simulink library "inp"  (Simulink 8.2)
settings: Solver=ode45  SolverMode=SingleTasking  StartTime=0.0  StopTime=10.0  RelTol=1e-3
undefined symbols (not defined in the file or in its folder): C_TICK_TIME_S, ENUM_FAULTSTATE_FAIL, ENUM_FAULTSTATE_INDETERMINATE, ENUM_FAULTSTATE_PASS, ENUM_KEYSWITCH_ON, FALSE, KINP_ABS1CANCommuOk_flg_ovrdflg, KINP_ABS1CANCommuOk_flg_ovrdval, KINP_ABS1CANPeriodTime_s, KINP_ABS1CANRolCntOk_flg_ovrdflg, KINP_ABS1CANRolCntOk_flg_ovrdval, KINP_ABS2CANChkSumOk_flg_ovrdflg, KINP_ABS2CANChkSumOk_flg_ovrdval, KINP_ABS2CANCommuOk_flg_ovrdflg, KINP_ABS2CANCommuOk_flg_ovrdval, KINP_ABS2CANPeriodTime_s, KINP_ABS2CANRolCntOk_flg_ovrdflg, KINP_ABS2CANRolCntOk_flg_ovrdval, KINP_ABS3CANCommuOk_flg_ovrdflg, KINP_ABS3CANCommuOk_flg_ovrdval, KINP_ABS4CANCommuOk_flg_ovrdflg, KINP_ABS4CANCommuOk_flg_ovrdval, KINP_ABSActive_flg_defval, KINP_ABSActive_flg_invalid, KINP_ABSActive_flg_ovrdflg, KINP_ABSActive_flg_ovrdval, KINP_ABSActive_flg_setvalid, KINP_ABSActive_flg_srvcflg, KINP_ABSActive_flg_srvcval, KINP_ABSCANAlive_flg_ovrdflg, KINP_ABSCANAlive_flg_ovrdval, KINP_ABSCANCommuOk_flg_ovrdflg, KINP_ABSCANCommuOk_flg_ovrdval, KINP_ABSCANValidOk_flg_ovrdflg, KINP_ABSCANValidOk_flg_ovrdval, KINP_ABSFault_flg_defval, KINP_ABSFault_flg_invalid, KINP_ABSFault_flg_ovrdflg, KINP_ABSFault_flg_ovrdval, KINP_ABSFault_flg_setvalid, KINP_ABSFault_flg_srvcflg, KINP_ABSFault_flg_srvcval, KINP_AC1CANCommuOk_flg_ovrdflg, KINP_AC1CANCommuOk_flg_ovrdval, KINP_ACCANAlive_flg_ovrdflg, KINP_ACCANAlive_flg_ovrdval, KINP_ACCANCommuOk_flg_ovrdflg, KINP_ACCANCommuOk_flg_ovrdval, KINP_ACCANValidOk_flg_ovrdflg, KINP_ACCANValidOk_flg_ovrdval, KINP_ACCCancelH_flg_defval, KINP_ACCCancelH_flg_invalid, KINP_ACCCancelH_flg_ovrdflg, KINP_ACCCancelH_flg_ovrdval, KINP_ACCCancelH_flg_setvalid, KINP_ACCCancelH_flg_srvcflg, KINP_ACCCancelH_flg_srvcval, KINP_AccelPedalPosn_pct_defval, KINP_AccelPedalPosn_pct_gain, KINP_AccelPedalPosn_pct_invalid ... (+1866)
libraries: inp x192, hcu_lib x34, simulink x33
(part 2 of 21)

== system inp/INP/BCM_signals ==
  BHAL_outputs : Inport  OutDataTypeStr=Bus: BHAL_outputs
  CANvalid_bus : Inport  Port=2
  AnyDoorOpen : Subsystem
      settings: RTWSystemCode=Inline
  Bus Selector1 : BusSelector  OutputSignals=VHAL_KeySwitchStatus_enum
  Bus Selector{2,5,7..8,10..11,13,15,17,19} (10 alike) : BusSelector  OutputSignals=VINP_CANMsgValidBCM1_flg
  Bus Selector12 : BusSelector  OutputSignals=VHAL_LRDoorOpen_flg
  Bus Selector14 : BusSelector  OutputSignals=VHAL_RRDoorOpen_flg
  Bus Selector16 : BusSelector  OutputSignals=VHAL_TailGateOpen_flg
  Bus Selector18 : BusSelector  OutputSignals=VHAL_AnyDoorOpen_flg
  Bus Selector20 : BusSelector  OutputSignals=VHAL_HazardLightSw_flg
  Bus Selector21 : BusSelector  OutputSignals=VHAL_VehCrashed_flg,VHAL_VehCrashedInvalid_flg
  Bus Selector3 : BusSelector  OutputSignals=VHAL_TurnSignalSwL_flg
  Bus Selector32 : BusSelector  OutputSignals=VINP_CANMsgValidBCM2_flg
  Bus Selector4 : BusSelector  OutputSignals=VHAL_TurnSignalSwR_flg
  Bus Selector6 : BusSelector  OutputSignals=VHAL_DriverDoorOpen_flg
  Bus Selector9 : BusSelector  OutputSignals=VHAL_PassengerDoorOpen_flg
  Data Store Read{1,38,40,50..56} (10 alike) : DataStoreRead  DataStoreName=VDDML_BCM1CANCommuFault_AF_flg
  Data Store Read42 : DataStoreRead  DataStoreName=VDDML_BCM2CANCommuFault_AF_flg
  Data Store Read44 : DataStoreRead  DataStoreName=VDDML_CANVehCrashdSigFault_AF_flg
  DriverDoorOpen : Subsystem
      settings: RTWSystemCode=Inline
  HazardLightSw : Subsystem
      settings: RTWSystemCode=Inline
  KeySwitchStatus : Subsystem
      settings: RTWSystemCode=Inline
  LRDoorOpen : Subsystem
      settings: RTWSystemCode=Inline
  Logical Operator6 : Logic  Tag=struct('inputs','1','comment',struct('text','','placement',1),'logdata',struct('maxnumvals',inf,'plotchannels',[],'stepsize',0,'downsampling',1,'loggingmode',3,'channels',1),'operator','NOT','output',struct('class','default','type','Bool8','description','','address','','unit','','name','$S_$B'),'date',731430.46749816)  Operator=NOT  Inputs=1
  Logical Operator9 : Logic  Tag=struct('inputs','1','comment',struct('text','','placement',1),'logdata',struct('maxnumvals',inf,'plotchannels',[],'stepsize',0,'downsampling',1,'loggingmode',3,'channels',1),'operator','NOT','output',struct('class','default','type','Bool8','description','','address','','unit','','name','$S_$B'),'date',731430.46749816)  Operator=OR
  PassengerDoorOpen : Subsystem
      settings: RTWSystemCode=Inline
  RRDoorOpen : Subsystem
      settings: RTWSystemCode=Inline
  TailGateOpen : Subsystem
      settings: RTWSystemCode=Inline
  TurnSignalSwLeft : Subsystem
      settings: RTWSystemCode=Inline
  TurnSignalSwRight : Subsystem
      settings: RTWSystemCode=Inline
  VehCrashed : Subsystem
      settings: RTWSystemCode=Inline
  inputs_bus2 : BusCreator  Inputs=23
  BCM_signal_bus : Outport
  wires:
  inputs_bus2/1 -> BCM_signal_bus/1
  BHAL_outputs/1 -> [BHAL_outputs]
  Bus Selector2/1 -> KeySwitchStatus/2(msg_valid)  "<VINP_CANMsgValidBCM1_flg>"
  [CANvalid_bus] -> Bus Selector2/1
  Bus Selector1/1 -> KeySwitchStatus/1(signal_in)  "<VHAL_KeySwitchStatus_enum>"
  [BHAL_outputs] -> Bus Selector1/1
  Data Store Read52/1 -> KeySwitchStatus/3(Active_fault_flg)
  KeySwitchStatus/1(signal_out) -> inputs_bus2/1  "VINP_KeySwitchStatus_enum_InHAL"
  KeySwitchStatus/2(signal_valid_out) -> inputs_bus2/2  "VINP_KeySwitchStatusValid_flg_InHAL"
  Bus Selector5/1 -> TurnSignalSwLeft/2(msg_valid)  "<VINP_CANMsgValidBCM1_flg>"
  [CANvalid_bus] -> Bus Selector5/1
  Bus Selector3/1 -> TurnSignalSwLeft/1(signal_in)  "<VHAL_TurnSignalSwL_flg>"
  [BHAL_outputs] -> Bus Selector3/1
  TurnSignalSwLeft/1(signal_out) -> inputs_bus2/3  "VINP_TurnSignalSwL_flg_InHAL"
  TurnSignalSwLeft/2(signal_valid_out) -> inputs_bus2/4  "VINP_TurnSignalSwLValid_flg_InHAL"
  Bus Selector8/1 -> TurnSignalSwRight/2(msg_valid)  "<VINP_CANMsgValidBCM1_flg>"
  [CANvalid_bus] -> Bus Selector8/1
  Bus Selector4/1 -> TurnSignalSwRight/1(signal_in)  "<VHAL_TurnSignalSwR_flg>"
  [BHAL_outputs] -> Bus Selector4/1
  TurnSignalSwRight/1(signal_out) -> inputs_bus2/5  "VINP_TurnSignalSwR_flg_InHAL"
  TurnSignalSwRight/2(signal_valid_out) -> inputs_bus2/6  "VINP_TurnSignalSwRValid_flg_InHAL"
  CANvalid_bus/1 -> [CANvalid_bus]
  Data Store Read38/1 -> TurnSignalSwLeft/3(Active_fault_flg)
  Data Store Read50/1 -> TurnSignalSwRight/3(Active_fault_flg)
  Bus Selector7/1 -> DriverDoorOpen/2(msg_valid)  "<VINP_CANMsgValidBCM1_flg>"
  [CANvalid_bus] -> Bus Selector7/1
  Bus Selector6/1 -> DriverDoorOpen/1(signal_in)  "<VHAL_DriverDoorOpen_flg>"
  [BHAL_outputs] -> Bus Selector6/1
  DriverDoorOpen/1(signal_out) -> inputs_bus2/9  "VINP_DriverDoorOpen_flg_InHAL"
  DriverDoorOpen/2(signal_valid_out) -> inputs_bus2/10  "VINP_DriverDoorOpenValid_flg_InHAL"
  Data Store Read51/1 -> DriverDoorOpen/3(Active_fault_flg)
  Bus Selector10/1 -> PassengerDoorOpen/2(msg_valid)  "<VINP_CANMsgValidBCM1_flg>"
  [CANvalid_bus] -> Bus Selector10/1
  Bus Selector9/1 -> PassengerDoorOpen/1(signal_in)  "<VHAL_PassengerDoorOpen_flg>"
  [BHAL_outputs] -> Bus Selector9/1
  PassengerDoorOpen/1(signal_out) -> inputs_bus2/11  "VINP_PassengerDoorOpen_flg_InHAL"
  PassengerDoorOpen/2(signal_valid_out) -> inputs_bus2/12  "VINP_PassengerDoorOpenValid_flg_InHAL"
  Data Store Read53/1 -> PassengerDoorOpen/3(Active_fault_flg)
  Bus Selector11/1 -> LRDoorOpen/2(msg_valid)  "<VINP_CANMsgValidBCM1_flg>"
  [CANvalid_bus] -> Bus Selector11/1
  Bus Selector12/1 -> LRDoorOpen/1(signal_in)  "<VHAL_LRDoorOpen_flg>"
  [BHAL_outputs] -> Bus Selector12/1
  LRDoorOpen/1(signal_out) -> inputs_bus2/13  "VINP_LRDoorOpen_flg_InHAL"
  LRDoorOpen/2(signal_valid_out) -> inputs_bus2/14  "VINP_LRDoorOpenValid_flg_InHAL"
  Data Store Read54/1 -> LRDoorOpen/3(Active_fault_flg)
  Bus Selector13/1 -> RRDoorOpen/2(msg_valid)  "<VINP_CANMsgValidBCM1_flg>"
  [CANvalid_bus] -> Bus Selector13/1
  Bus Selector14/1 -> RRDoorOpen/1(signal_in)  "<VHAL_RRDoorOpen_flg>"
  [BHAL_outputs] -> Bus Selector14/1
  RRDoorOpen/1(signal_out) -> inputs_bus2/15  "VINP_RRDoorOpen_flg_InHAL"
  RRDoorOpen/2(signal_valid_out) -> inputs_bus2/16  "VINP_RRDoorOpenValid_flg_InHAL"
  Data Store Read55/1 -> RRDoorOpen/3(Active_fault_flg)
  Bus Selector15/1 -> TailGateOpen/2(msg_valid)  "<VINP_CANMsgValidBCM1_flg>"
  [CANvalid_bus] -> Bus Selector15/1
  Bus Selector16/1 -> TailGateOpen/1(signal_in)  "<VHAL_TailGateOpen_flg>"
  [BHAL_outputs] -> Bus Selector16/1
  TailGateOpen/1(signal_out) -> inputs_bus2/17  "VINP_TailGateOpen_flg_InHAL"
  TailGateOpen/2(signal_valid_out) -> inputs_bus2/18  "VINP_TailGateOpenValid_flg_InHAL"
  Data Store Read1/1 -> TailGateOpen/3(Active_fault_flg)
  Bus Selector17/1 -> AnyDoorOpen/2(msg_valid)  "<VINP_CANMsgValidBCM1_flg>"
  [CANvalid_bus] -> Bus Selector17/1
  Bus Selector18/1 -> AnyDoorOpen/1(signal_in)  "<VHAL_AnyDoorOpen_flg>"
  [BHAL_outputs] -> Bus Selector18/1
  AnyDoorOpen/1(signal_out) -> inputs_bus2/19  "VINP_AnyDoorOpen_flg_InHAL"
  AnyDoorOpen/2(signal_valid_out) -> inputs_bus2/20  "VINP_AnyDoorOpenValid_flg_InHAL"
  Data Store Read56/1 -> AnyDoorOpen/3(Active_fault_flg)
  Bus Selector19/1 -> HazardLightSw/2(msg_valid)  "<VINP_CANMsgValidBCM1_flg>"
  [CANvalid_bus] -> Bus Selector19/1
  Bus Selector20/1 -> HazardLightSw/1(signal_in)  "<VHAL_HazardLightSw_flg>"
  [BHAL_outputs] -> Bus Selector20/1
  HazardLightSw/1(signal_out) -> inputs_bus2/7  "VINP_HazardLightSw_flg_InHAL"
  HazardLightSw/2(signal_valid_out) -> inputs_bus2/8  "VINP_HazardLightSwValid_flg_InHAL"
  Data Store Read40/1 -> HazardLightSw/3(Active_fault_flg)
  Bus Selector32/1 -> VehCrashed/3(msg_valid)  "<VINP_CANMsgValidBCM2_flg>"
  [CANvalid_bus] -> Bus Selector32/1
  Logical Operator6/1 -> VehCrashed/2(signal_valid_in)
  Bus Selector21/2 -> Logical Operator6/1  "<VHAL_VehCrashedInvalid_flg>"
  Bus Selector21/1 -> VehCrashed/1(signal_in)  "<VHAL_VehCrashed_flg>"
  [BHAL_outputs] -> Bus Selector21/1
  VehCrashed/1(signal_out) -> inputs_bus2/21  "VINP_VehCrashed_flg_InHAL"
  VehCrashed/2(signal_valid_out) -> inputs_bus2/22  "VINP_VehCrashedValid_flg_InHAL"
  VehCrashed/3(raw_signal_valid_out) -> inputs_bus2/23  "VINP_VehCrashedSigOk_flg_InHAL"
  Logical Operator9/1 -> VehCrashed/4(Active_fault_flg)
  Data Store Read44/1 -> Logical Operator9/1
  Data Store Read42/1 -> Logical Operator9/2

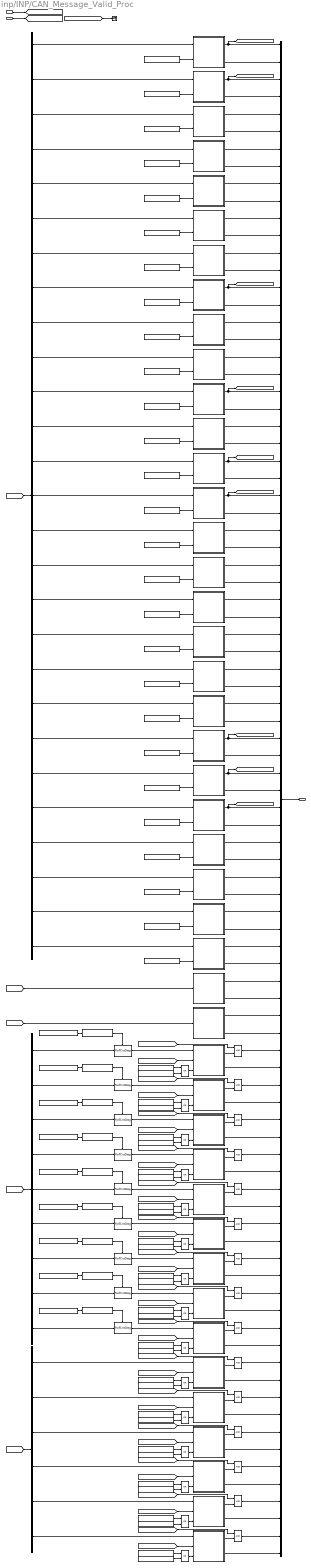
== system inp/INP/CAN_Message_Valid_Proc ==
  BHAL_outputs : Inport
  Task_Time_s : Inport  Port=2
  ABS1Updated : Subsystem
      settings: RTWSystemCode=Inline
  ABS1Valid : Subsystem
      settings: RTWSystemCode=Inline
  ABS2Checked : Subsystem
      settings: RTWSystemCode=Inline
  ABS2Updated : Subsystem
      settings: RTWSystemCode=Inline
  ABS2Valid : Subsystem
      settings: RTWSystemCode=Inline
  ABS3Valid : Subsystem
      settings: RTWSystemCode=Inline
  ABS4Valid : Subsystem
      settings: RTWSystemCode=Inline
  AC1Valid : Subsystem
      settings: RTWSystemCode=Inline
  BCM1Valid : Subsystem
      settings: RTWSystemCode=Inline
  BCM2Valid : Subsystem
      settings: RTWSystemCode=Inline
  Bus Selector : BusSelector  OutputSignals=VHAL_ABS2ChkSumValid_flg,VHAL_SAS1ChkSumValid_flg,VHAL_EPAS2ChkSumValid_flg,VHAL_EPAS3ChkSumValid_flg,VHAL_VCU1ChkSumValid_flg,VHAL_VCU2ChkSumValid_flg
  Bus Selector1 : BusSelector  OutputSignals=VHAL_ECM9RollCnt_cnt,VHAL_ABS1RollCnt_cnt,VHAL_ABS2RollCnt_cnt,VHAL_SAS1RollCnt_cnt,VHAL_EPAS2RollCnt_cnt,VHAL_EPAS3RollCnt_cnt,VHAL_VCU1RollCnt_cnt,VHAL_VCU2RollCnt_cnt,VHAL_HCU3RollCnt_cnt
  Bus Selector5 : BusSelector  OutputSignals=VHAL_CANMsgValidABS1_flg,VHAL_CANMsgValidABS2_flg,VHAL_CANMsgValidABS3_flg,VHAL_CANMsgValidABS4_flg,VHAL_CANMsgValidECM1_flg,VHAL_CANMsgValidECM2_flg,VHAL_CANMsgValidECM3_flg,VHAL_CANMsgValidECM9_flg,VHAL_CANMsgValidTCM1_flg,VHAL_CANMsgValidTCM2_flg,VHAL_CANMsgValidSAS1_flg,VHAL_CANMsgValidEPAS1_flg,VHAL_CANMsgValidEPAS2_flg,VHAL_CANMsgValidEPAS3_flg,VHAL_CANMsgValidBCM1_flg,VHAL_CANMsgValidBCM2_flg,VHAL_CANMsgValidMETER1_flg,VHAL_CANMsgValidMETER3_flg,VHAL_CANMsgValidAC1_flg,VHAL_CANMsgValidEPB1_flg,VHAL_CANMsgValidVCU1_flg,VHAL_CANMsgValidVCU2_flg,VHAL_CANMsgValidHCU3_flg,VHAL_CANMsgValidHCU4_flg,VHAL_CANMsgValidHMI2_flg,VHAL_CANMsgValidHMI1_flg,VHAL_CANMsgValidCSW1_flg
  CSW1Valid : Subsystem
      settings: RTWSystemCode=Inline
  Constant1 : Constant  Value=KINP_ECM9CANPeriodTime_s
  Constant2 : Constant  Value=KINP_EPAS2CANPeriodTime_s
  Constant3 : Constant  Value=KINP_ABS1CANPeriodTime_s
  Constant4 : Constant  Value=KINP_SAS1CANPeriodTime_s
  Constant5 : Constant  Value=KINP_EPAS3CANPeriodTime_s
  Constant6 : Constant  Value=KINP_ABS2CANPeriodTime_s
  Constant7 : Constant  Value=KINP_VCU1CANPeriodTime_s
  Constant8 : Constant  Value=KINP_VCU2CANPeriodTime_s
  Constant9 : Constant  Value=KINP_HCU3CANPeriodTime_s
  Data Store Read : DataStoreRead  DataStoreName=VDDML_ABS2CANCommuFault_AF_flg
  Data Store Read1 : DataStoreRead  DataStoreName=VDDML_ABS4CANCommuFault_AF_flg
  Data Store Read10 : DataStoreRead  DataStoreName=VDDML_SAS1CANCommuFault_AF_flg
  Data Store Read11 : DataStoreRead  DataStoreName=VDDML_ECM9CANCommuFault_AF_flg
  Data Store Read12 : DataStoreRead  DataStoreName=VDDML_ECM9CANRolCntFault_AF_flg
  Data Store Read13 : DataStoreRead  DataStoreName=VDDML_SAS1CANCommuFault_AF_flg
  Data Store Read14 : DataStoreRead  DataStoreName=VDDML_SAS1CANRolCntFault_AF_flg
  Data Store Read15 : DataStoreRead  DataStoreName=VDDML_ABS2CANCommuFault_AF_flg
  Data Store Read16 : DataStoreRead  DataStoreName=VDDML_ABS2CANRolCntFault_AF_flg
  Data Store Read17 : DataStoreRead  DataStoreName=VDDML_EPAS2CANCommuFault_AF_flg
  Data Store Read18 : DataStoreRead  DataStoreName=VDDML_EPAS3CANCommuFault_AF_flg
  Data Store Read19 : DataStoreRead  DataStoreName=VDDML_EPAS2CANCommuFault_AF_flg
  Data Store Read2 : DataStoreRead  DataStoreName=VDDML_BCM1CANCommuFault_AF_flg
  Data Store Read20 : DataStoreRead  DataStoreName=VDDML_EPAS2CANRolCntFault_AF_flg
  Data Store Read21 : DataStoreRead  DataStoreName=VDDML_EPAS3CANCommuFault_AF_flg
  Data Store Read22 : DataStoreRead  DataStoreName=VDDML_EPAS3CANRolCntFault_AF_flg
  Data Store Read23 : DataStoreRead  DataStoreName=VDDML_METER1CANCommuFault_AF_flg
  Data Store Read24 : DataStoreRead  DataStoreName=VDDML_ECM1CANCommuFault_AF_flg
  Data Store Read25 : DataStoreRead  DataStoreName=VDDML_ABS1CANCommuFault_AF_flg
  Data Store Read26 : DataStoreRead  DataStoreName=VDDML_METER3CANCommuFault_AF_flg
  Data Store Read27 : DataStoreRead  DataStoreName=VDDML_AC1CANCommuFault_AF_flg
  Data Store Read28 : DataStoreRead  DataStoreName=VDDML_EPB1CANCommuFault_AF_flg
  Data Store Read29 : DataStoreRead  DataStoreName=VDDML_VCU1CANCommuFault_AF_flg
  Data Store Read3 : DataStoreRead  DataStoreName=VDDML_ECM3CANCommuFault_AF_flg
  Data Store Read30 : DataStoreRead  DataStoreName=VDDML_VCU2CANCommuFault_AF_flg
  Data Store Read31 : DataStoreRead  DataStoreName=VDDML_HCU3CANCommuFault_AF_flg
  Data Store Read32 : DataStoreRead  DataStoreName=VDDML_ABS1CANCommuFault_AF_flg
  Data Store Read33 : DataStoreRead  DataStoreName=VDDML_ABS1CANRolCntFault_AF_flg
  Data Store Read34 : DataStoreRead  DataStoreName=VDDML_VCU1CANCommuFault_AF_flg
  Data Store Read35 : DataStoreRead  DataStoreName=VDDML_VCU1CANRolCntFault_AF_flg
  Data Store Read36 : DataStoreRead  DataStoreName=VDDML_VCU2CANCommuFault_AF_flg
  Data Store Read37 : DataStoreRead  DataStoreName=VDDML_VCU2CANRolCntFault_AF_flg
  Data Store Read38 : DataStoreRead  DataStoreName=VDDML_ABS2CANCommuFault_AF_flg
  Data Store Read39 : DataStoreRead  DataStoreName=VDDML_ABS2CANChkSumFault_AF_flg
  Data Store Read4 : DataStoreRead  DataStoreName=VDDML_ECM9CANCommuFault_AF_flg
  Data Store Read40 : DataStoreRead  DataStoreName=VDDML_SAS1CANCommuFault_AF_flg
  Data Store Read41 : DataStoreRead  DataStoreName=VDDML_SAS1CANChkSumFault_AF_flg
  Data Store Read42 : DataStoreRead  DataStoreName=VDDML_EPAS2CANCommuFault_AF_flg
  Data Store Read43 : DataStoreRead  DataStoreName=VDDML_EPAS2CANChkSumFault_AF_flg
  Data Store Read44 : DataStoreRead  DataStoreName=VDDML_EPAS3CANCommuFault_AF_flg
  Data Store Read45 : DataStoreRead  DataStoreName=VDDML_EPAS3CANChkSumFault_AF_flg
  Data Store Read46 : DataStoreRead  DataStoreName=VDDML_VCU1CANCommuFault_AF_flg
  Data Store Read47 : DataStoreRead  DataStoreName=VDDML_VCU1CANChkSumFault_AF_flg
  Data Store Read48 : DataStoreRead  DataStoreName=VDDML_VCU2CANCommuFault_AF_flg
  Data Store Read49 : DataStoreRead  DataStoreName=VDDML_VCU2CANChkSumFault_AF_flg
  Data Store Read5 : DataStoreRead  DataStoreName=VDDML_TCM1CANCommuFault_AF_flg
  Data Store Read50 : DataStoreRead  DataStoreName=VDDML_HCU4CANCommuFault_AF_flg
  Data Store Read51 : DataStoreRead  DataStoreName=VDDML_HMI1CANCommuFault_AF_flg
  Data Store Read52 : DataStoreRead  DataStoreName=VDDML_HMI2CANCommuFault_AF_flg
  Data Store Read58 : DataStoreRead  DataStoreName=VDDML_HCU3CANCommuFault_AF_flg
  Data Store Read59 : DataStoreRead  DataStoreName=VDDML_HCU3CANRolCntFault_AF_flg
  Data Store Read6 : DataStoreRead  DataStoreName=VDDML_ABS3CANCommuFault_AF_flg
  Data Store Read60 : DataStoreRead  DataStoreName=VDDML_EPAS1CANCommuFault_AF_flg
  Data Store Read61 : DataStoreRead  DataStoreName=VDDML_CSW1CANCommuFault_AF_flg
  Data Store Read7 : DataStoreRead  DataStoreName=VDDML_ECM2CANCommuFault_AF_flg
  Data Store Read8 : DataStoreRead  DataStoreName=VDDML_TCM2CANCommuFault_AF_flg
  Data Store Read9 : DataStoreRead  DataStoreName=VDDML_BCM2CANCommuFault_AF_flg
  ECM1Valid : Subsystem
      settings: RTWSystemCode=Inline
  ECM2Valid : Subsystem
      settings: RTWSystemCode=Inline
  ECM3Valid : Subsystem
      settings: RTWSystemCode=Inline
  ECM9Updated : Subsystem
      settings: RTWSystemCode=Inline
  ECM9Valid : Subsystem
      settings: RTWSystemCode=Inline
  EPAS1Valid : Subsystem
      settings: RTWSystemCode=Inline
  EPAS2Checked : Subsystem
      settings: RTWSystemCode=Inline
  EPAS2Updated : Subsystem
      settings: RTWSystemCode=Inline
  EPAS2Valid : Subsystem
      settings: RTWSystemCode=Inline
  EPAS3Checked : Subsystem
      settings: RTWSystemCode=Inline
  EPAS3Updated : Subsystem
      settings: RTWSystemCode=Inline
  EPAS3Valid : Subsystem
      settings: RTWSystemCode=Inline
  EPB1Valid : Subsystem
      settings: RTWSystemCode=Inline
  EPMCheckReceived : Subsystem
  EPMValid : Subsystem
  Function Call Generator ABS1 : hcu_lib/Function Call Generator  SystemSampleTime=-1
  Function Call Generator ABS2 : hcu_lib/Function Call Generator  SystemSampleTime=-1
  Function Call Generator ECM9 : hcu_lib/Function Call Generator  SystemSampleTime=-1
  Function Call Generator EPAS2 : hcu_lib/Function Call Generator  SystemSampleTime=-1
  Function Call Generator EPAS3 : hcu_lib/Function Call Generator  SystemSampleTime=-1
  Function Call Generator HCU3 : hcu_lib/Function Call Generator  SystemSampleTime=-1
  Function Call Generator SAS1 : hcu_lib/Function Call Generator  SystemSampleTime=-1
  Function Call Generator VCU1 : hcu_lib/Function Call Generator  SystemSampleTime=-1
  Function Call Generator VCU2 : hcu_lib/Function Call Generator  SystemSampleTime=-1
  HCU3Updated : Subsystem
      settings: RTWSystemCode=Inline
  HCU3Valid : Subsystem
      settings: RTWSystemCode=Inline
  HCU4Valid : Subsystem
      settings: RTWSystemCode=Inline
  HMI1Valid : Subsystem
      settings: RTWSystemCode=Inline
  HMI2Valid : Subsystem
      settings: RTWSystemCode=Inline
  Logical Operator{1,4..5,8,10..11,13,15,17,20,22..23,25,27,29} (15 alike) : Logic  AllPortsSameDT=off  OutDataTypeStr=boolean
  Logical Operator{2..3,6..7,9,12,14,16,18..19,21,24,26,28,30} (15 alike) : Logic  Tag=struct('inputs','1','comment',struct('text','','placement',1),'logdata',struct('maxnumvals',inf,'plotchannels',[],'stepsize',0,'downsampling',1,'loggingmode',3,'channels',1),'operator','NOT','output',struct('class','default','type','Bool8','description','','address','','unit','','name','$S_$B'),'date',731430.46749816)  Operator=OR
  METER1Valid : Subsystem
      settings: RTWSystemCode=Inline
  METER3Valid : Subsystem
      settings: RTWSystemCode=Inline
  RollCntDiag_ABS1 : inp/RollCntDiag
  RollCntDiag_ABS2 : inp/RollCntDiag
  RollCntDiag_ECM9 : inp/RollCntDiag
  RollCntDiag_EPAS2 : inp/RollCntDiag
  RollCntDiag_EPAS3 : inp/RollCntDiag
  RollCntDiag_HCU3 : inp/RollCntDiag
  RollCntDiag_SAS1 : inp/RollCntDiag
  RollCntDiag_VCU1 : inp/RollCntDiag
  RollCntDiag_VCU2 : inp/RollCntDiag
  SAS1Checked : Subsystem
      settings: RTWSystemCode=Inline
  SAS1Updated : Subsystem
      settings: RTWSystemCode=Inline
  SAS1Valid : Subsystem
      settings: RTWSystemCode=Inline
  TCM1Valid : Subsystem
      settings: RTWSystemCode=Inline
  TCM2Valid : Subsystem
      settings: RTWSystemCode=Inline
  Terminator : Terminator
  VCU1Checked : Subsystem
      settings: RTWSystemCode=Inline
  VCU1Updated : Subsystem
      settings: RTWSystemCode=Inline
  VCU1Valid : Subsystem
      settings: RTWSystemCode=Inline
  VCU2Checked : Subsystem
      settings: RTWSystemCode=Inline
  VCU2Updated : Subsystem
      settings: RTWSystemCode=Inline
  VCU2Valid : Subsystem
      settings: RTWSystemCode=Inline
  inputs_bus2 : BusCreator  Inputs=88
  CANvalid_bus : Outport
  wires:
  inputs_bus2/1 -> CANvalid_bus/1
  Logical Operator5/1 -> inputs_bus2/65  "VINP_CANMsgUpdatedSAS1_flg"
  Logical Operator4/1 -> inputs_bus2/59  "VINP_CANMsgUpdatedECM9_flg"
  RollCntDiag_SAS1/1 -> SAS1Updated/1(signal_in)
  RollCntDiag_ECM9/1 -> ECM9Updated/1(signal_in)
  BHAL_outputs/1 -> [BHAL_outputs]
  Bus Selector1/4 -> RollCntDiag_SAS1/1  "<VHAL_SAS1RollCnt_cnt>"
  Bus Selector1/1 -> RollCntDiag_ECM9/1  "<VHAL_ECM9RollCnt_cnt>"
  Task_Time_s/1 -> [Task_Time_s]
  [BHAL_outputs] -> Bus Selector1/1
  Function Call Generator ECM9/1 -> RollCntDiag_ECM9/Trigger
  Constant1/1 -> Function Call Generator ECM9/1
  Constant4/1 -> Function Call Generator SAS1/1
  Function Call Generator SAS1/1 -> RollCntDiag_SAS1/Trigger
  RollCntDiag_ABS2/1 -> ABS2Updated/1(signal_in)
  Constant6/1 -> Function Call Generator ABS2/1
  Function Call Generator ABS2/1 -> RollCntDiag_ABS2/Trigger
  Logical Operator1/1 -> inputs_bus2/63  "VINP_CANMsgUpdatedABS2_flg"
  ECM9Updated/1(signal_out) -> Logical Operator4/2
  ECM9Updated/2(signal_valid_out) -> inputs_bus2/60  "VINP_CANMsgECM9RolCntOk_flg"
  Data Store Read12/1 -> Logical Operator2/1
  SAS1Updated/1(signal_out) -> Logical Operator5/2
  SAS1Updated/2(signal_valid_out) -> inputs_bus2/66  "VINP_CANMsgSAS1RolCntOk_flg"
  ABS2Updated/1(signal_out) -> Logical Operator1/2
  ABS2Updated/2(signal_valid_out) -> inputs_bus2/64  "VINP_CANMsgABS2RolCntOk_flg"
  [BHAL_outputs] -> Bus Selector5/1
  Data Store Read/1 -> ABS2Valid/2(Active_fault_flg)
  ABS2Valid/1(signal_out) -> inputs_bus2/3, [VINP_CANMsgValidABS2_flg]  "VINP_CANMsgValidABS2_flg"
  ABS2Valid/2(signal_valid_out) -> inputs_bus2/4  "VINP_CANMsgABS2CommuOk_flg"
  ABS4Valid/1(signal_out) -> inputs_bus2/7  "VINP_CANMsgValidABS4_flg"
  ABS4Valid/2(signal_valid_out) -> inputs_bus2/8  "VINP_CANMsgABS4CommuOk_flg"
  Bus Selector5/2 -> ABS2Valid/1(signal_in)  "<VHAL_CANMsgValidABS2_flg>"
  Bus Selector5/4 -> ABS4Valid/1(signal_in)  "<VHAL_CANMsgValidABS4_flg>"
  Data Store Read1/1 -> ABS4Valid/2(Active_fault_flg)
  Data Store Read2/1 -> BCM1Valid/2(Active_fault_flg)
  BCM1Valid/1(signal_out) -> inputs_bus2/29  "VINP_CANMsgValidBCM1_flg"
  BCM1Valid/2(signal_valid_out) -> inputs_bus2/30  "VINP_CANMsgBCM1CommuOk_flg"
  Bus Selector5/15 -> BCM1Valid/1(signal_in)  "<VHAL_CANMsgValidBCM1_flg>"
  ECM3Valid/1(signal_out) -> inputs_bus2/13  "VINP_CANMsgValidECM3_flg"
  ECM3Valid/2(signal_valid_out) -> inputs_bus2/14  "VINP_CANMsgECM3CommuOk_flg"
  Bus Selector5/7 -> ECM3Valid/1(signal_in)  "<VHAL_CANMsgValidECM3_flg>"
  ECM9Valid/1(signal_out) -> inputs_bus2/15, [VINP_CANMsgValidECM9_flg]  "VINP_CANMsgValidECM9_flg"
  Bus Selector5/8 -> ECM9Valid/1(signal_in)  "<VHAL_CANMsgValidECM9_flg>"
  ECM9Valid/2(signal_valid_out) -> inputs_bus2/16  "VINP_CANMsgECM9CommuOk_flg"
  TCM1Valid/1(signal_out) -> inputs_bus2/17  "VINP_CANMsgValidTCM1_flg"
  TCM1Valid/2(signal_valid_out) -> inputs_bus2/18  "VINP_CANMsgTCM1CommuOk_flg"
  Bus Selector5/9 -> TCM1Valid/1(signal_in)  "<VHAL_CANMsgValidTCM1_flg>"
  SAS1Valid/1(signal_out) -> inputs_bus2/21, [VINP_CANMsgValidSAS1_flg]  "VINP_CANMsgValidSAS1_flg"
  SAS1Valid/2(signal_valid_out) -> inputs_bus2/22  "VINP_CANMsgSAS1CommuOk_flg"
  Bus Selector5/11 -> SAS1Valid/1(signal_in)  "<VHAL_CANMsgValidSAS1_flg>"
  [VINP_CANMsgValidECM9_flg] -> Logical Operator4/1
  [VINP_CANMsgValidSAS1_flg] -> Logical Operator5/1
  [VINP_CANMsgValidABS2_flg] -> Logical Operator1/1
  [VINP_CANMsgValidECM9_flg] -> ECM9Updated/2(diagnosis_switch)
  [VINP_CANMsgValidSAS1_flg] -> SAS1Updated/2(diagnosis_switch)
  [VINP_CANMsgValidABS2_flg] -> ABS2Updated/2(diagnosis_switch)
  Logical Operator2/1 -> ECM9Updated/3(Active_fault_flg)
  Data Store Read11/1 -> Logical Operator2/2
  Data Store Read14/1 -> Logical Operator3/1
  Data Store Read13/1 -> Logical Operator3/2
  Logical Operator3/1 -> SAS1Updated/3(Active_fault_flg)
  Data Store Read16/1 -> Logical Operator6/1
  Data Store Read15/1 -> Logical Operator6/2
  Logical Operator6/1 -> ABS2Updated/3(Active_fault_flg)
  Data Store Read3/1 -> ECM3Valid/2(Active_fault_flg)
  Data Store Read4/1 -> ECM9Valid/2(Active_fault_flg)
  Data Store Read5/1 -> TCM1Valid/2(Active_fault_flg)
  Data Store Read10/1 -> SAS1Valid/2(Active_fault_flg)
  Bus Selector1/3 -> RollCntDiag_ABS2/1  "<VHAL_ABS2RollCnt_cnt>"
  Data Store Read17/1 -> EPAS2Valid/2(Active_fault_flg)
  EPAS2Valid/1(signal_out) -> inputs_bus2/25, [VINP_CANMsgValidEPAS2_flg]  "VINP_CANMsgValidEPAS2_flg"
  EPAS2Valid/2(signal_valid_out) -> inputs_bus2/26  "VINP_CANMsgEPAS2CommuOk_flg"
  Data Store Read18/1 -> EPAS3Valid/2(Active_fault_flg)
  Bus Selector5/14 -> EPAS3Valid/1(signal_in)  "<VHAL_CANMsgValidEPAS3_flg>"
  EPAS3Valid/1(signal_out) -> inputs_bus2/27, [VINP_CANMsgValidEPAS3_flg]  "VINP_CANMsgValidEPAS3_flg"
  EPAS3Valid/2(signal_valid_out) -> inputs_bus2/28  "VINP_CANMsgEPAS3CommuOk_flg"
  Logical Operator8/1 -> inputs_bus2/67  "VINP_CANMsgUpdatedEPAS2_flg"
  RollCntDiag_EPAS2/1 -> EPAS2Updated/1(signal_in)
  Constant2/1 -> Function Call Generator EPAS2/1
  EPAS2Updated/1(signal_out) -> Logical Operator8/2
  EPAS2Updated/2(signal_valid_out) -> inputs_bus2/68  "VINP_CANMsgEPAS2RolCntOk_flg"
  [VINP_CANMsgValidEPAS2_flg] -> Logical Operator8/1
  [VINP_CANMsgValidEPAS2_flg] -> EPAS2Updated/2(diagnosis_switch)
  Data Store Read20/1 -> Logical Operator7/1
  Data Store Read19/1 -> Logical Operator7/2
  Logical Operator7/1 -> EPAS2Updated/3(Active_fault_flg)
  Bus Selector1/5 -> RollCntDiag_EPAS2/1  "<VHAL_EPAS2RollCnt_cnt>"
  Function Call Generator EPAS2/1 -> RollCntDiag_EPAS2/Trigger
  RollCntDiag_EPAS3/1 -> EPAS3Updated/1(signal_in)
  Constant5/1 -> Function Call Generator EPAS3/1
  EPAS3Updated/1(signal_out) -> Logical Operator10/2
  [VINP_CANMsgValidEPAS3_flg] -> Logical Operator10/1
  [VINP_CANMsgValidEPAS3_flg] -> EPAS3Updated/2(diagnosis_switch)
  Data Store Read22/1 -> Logical Operator9/1
  Data Store Read21/1 -> Logical Operator9/2
  Logical Operator9/1 -> EPAS3Updated/3(Active_fault_flg)
  Function Call Generator EPAS3/1 -> RollCntDiag_EPAS3/Trigger
  Bus Selector1/6 -> RollCntDiag_EPAS3/1  "<VHAL_EPAS3RollCnt_cnt>"
  Logical Operator10/1 -> inputs_bus2/69  "VINP_CANMsgUpdatedEPAS3_flg"
  EPAS3Updated/2(signal_valid_out) -> inputs_bus2/70  "VINP_CANMsgEPAS3RolCntOk_flg"
  Bus Selector5/13 -> EPAS2Valid/1(signal_in)  "<VHAL_CANMsgValidEPAS2_flg>"
  METER1Valid/1(signal_out) -> inputs_bus2/33  "VINP_CANMsgValidMETER1_flg"
  METER1Valid/2(signal_valid_out) -> inputs_bus2/34  "VINP_CANMsgMETER1CommuOk_flg"
  Bus Selector5/17 -> METER1Valid/1(signal_in)  "<VHAL_CANMsgValidMETER1_flg>"
  Data Store Read23/1 -> METER1Valid/2(Active_fault_flg)
  ECM1Valid/1(signal_out) -> inputs_bus2/9  "VINP_CANMsgValidECM1_flg"
  ECM1Valid/2(signal_valid_out) -> inputs_bus2/10  "VINP_CANMsgECM1CommuOk_flg"
  Bus Selector5/5 -> ECM1Valid/1(signal_in)  "<VHAL_CANMsgValidECM1_flg>"
  Data Store Read24/1 -> ECM1Valid/2(Active_fault_flg)
  Data Store Read25/1 -> ABS1Valid/2(Active_fault_flg)
  Bus Selector5/1 -> ABS1Valid/1(signal_in)  "<VHAL_CANMsgValidABS1_flg>"
  ABS1Valid/1(signal_out) -> inputs_bus2/1, [VINP_CANMsgValidABS1_flg]  "VINP_CANMsgValidABS1_flg"
  ABS1Valid/2(signal_valid_out) -> inputs_bus2/2  "VINP_CANMsgABS1CommuOk_flg"
  [Task_Time_s] -> Terminator/1
  Data Store Read6/1 -> ABS3Valid/2(Active_fault_flg)
  Bus Selector5/3 -> ABS3Valid/1(signal_in)  "<VHAL_CANMsgValidABS3_flg>"
  ABS3Valid/1(signal_out) -> inputs_bus2/5  "VINP_CANMsgValidABS3_flg"
  ABS3Valid/2(signal_valid_out) -> inputs_bus2/6  "VINP_CANMsgABS3CommuOk_flg"
  ECM2Valid/1(signal_out) -> inputs_bus2/11  "VINP_CANMsgValidECM2_flg"
  ECM2Valid/2(signal_valid_out) -> inputs_bus2/12  "VINP_CANMsgECM2CommuOk_flg"
  Bus Selector5/6 -> ECM2Valid/1(signal_in)  "<VHAL_CANMsgValidECM2_flg>"
  Data Store Read7/1 -> ECM2Valid/2(Active_fault_flg)
  TCM2Valid/1(signal_out) -> inputs_bus2/19  "VINP_CANMsgValidTCM2_flg"
  TCM2Valid/2(signal_valid_out) -> inputs_bus2/20  "VINP_CANMsgTCM2CommuOk_flg"
  Bus Selector5/10 -> TCM2Valid/1(signal_in)  "<VHAL_CANMsgValidTCM2_flg>"
  Data Store Read8/1 -> TCM2Valid/2(Active_fault_flg)
  Data Store Read9/1 -> BCM2Valid/2(Active_fault_flg)
  BCM2Valid/1(signal_out) -> inputs_bus2/31  "VINP_CANMsgValidBCM2_flg"
  BCM2Valid/2(signal_valid_out) -> inputs_bus2/32  "VINP_CANMsgBCM2CommuOk_flg"
  Bus Selector5/16 -> BCM2Valid/1(signal_in)  "<VHAL_CANMsgValidBCM2_flg>"
  METER3Valid/1(signal_out) -> inputs_bus2/35  "VINP_CANMsgValidMETER3_flg"
  METER3Valid/2(signal_valid_out) -> inputs_bus2/36  "VINP_CANMsgMETER3CommuOk_flg"
  Bus Selector5/18 -> METER3Valid/1(signal_in)  "<VHAL_CANMsgValidMETER3_flg>"
  Data Store Read26/1 -> METER3Valid/2(Active_fault_flg)
  AC1Valid/1(signal_out) -> inputs_bus2/37  "VINP_CANMsgValidAC1_flg"
  AC1Valid/2(signal_valid_out) -> inputs_bus2/38  "VINP_CANMsgAC1CommuOk_flg"
  Bus Selector5/19 -> AC1Valid/1(signal_in)  "<VHAL_CANMsgValidAC1_flg>"
  Data Store Read27/1 -> AC1Valid/2(Active_fault_flg)
  EPB1Valid/1(signal_out) -> inputs_bus2/39  "VINP_CANMsgValidEPB1_flg"
  EPB1Valid/2(signal_valid_out) -> inputs_bus2/40  "VINP_CANMsgEPB1CommuOk_flg"
  Bus Selector5/20 -> EPB1Valid/1(signal_in)  "<VHAL_CANMsgValidEPB1_flg>"
  Data Store Read28/1 -> EPB1Valid/2(Active_fault_flg)
  VCU1Valid/1(signal_out) -> inputs_bus2/41, [VINP_CANMsgValidVCU1_flg]  "VINP_CANMsgValidVCU1_flg"
  VCU1Valid/2(signal_valid_out) -> inputs_bus2/42  "VINP_CANMsgVCU1CommuOk_flg"
  Bus Selector5/21 -> VCU1Valid/1(signal_in)  "<VHAL_CANMsgValidVCU1_flg>"
  Data Store Read29/1 -> VCU1Valid/2(Active_fault_flg)
  VCU2Valid/1(signal_out) -> inputs_bus2/43, [VINP_CANMsgValidVCU2_flg]  "VINP_CANMsgValidVCU2_flg"
  VCU2Valid/2(signal_valid_out) -> inputs_bus2/44  "VINP_CANMsgVCU2CommuOk_flg"
  Bus Selector5/22 -> VCU2Valid/1(signal_in)  "<VHAL_CANMsgValidVCU2_flg>"
  Data Store Read30/1 -> VCU2Valid/2(Active_fault_flg)
  HCU3Valid/1(signal_out) -> inputs_bus2/45, [VINP_CANMsgValidHCU3_flg]  "VINP_CANMsgValidHCU3_flg"
  HCU3Valid/2(signal_valid_out) -> inputs_bus2/46  "VINP_CANMsgHCU3CommuOk_flg"
  Bus Selector5/23 -> HCU3Valid/1(signal_in)  "<VHAL_CANMsgValidHCU3_flg>"
  Data Store Read31/1 -> HCU3Valid/2(Active_fault_flg)
  RollCntDiag_ABS1/1 -> ABS1Updated/1(signal_in)
  Constant3/1 -> Function Call Generator ABS1/1
  Function Call Generator ABS1/1 -> RollCntDiag_ABS1/Trigger
  Logical Operator11/1 -> inputs_bus2/61  "VINP_CANMsgUpdatedABS1_flg"
  ABS1Updated/1(signal_out) -> Logical Operator11/2
  ABS1Updated/2(signal_valid_out) -> inputs_bus2/62  "VINP_CANMsgABS1RolCntOk_flg"
  [VINP_CANMsgValidABS1_flg] -> Logical Operator11/1
  [VINP_CANMsgValidABS1_flg] -> ABS1Updated/2(diagnosis_switch)
  Data Store Read33/1 -> Logical Operator12/1
  Data Store Read32/1 -> Logical Operator12/2
  Logical Operator12/1 -> ABS1Updated/3(Active_fault_flg)
  Bus Selector1/2 -> RollCntDiag_ABS1/1  "<VHAL_ABS1RollCnt_cnt>"
  RollCntDiag_VCU1/1 -> VCU1Updated/1(signal_in)
  Constant7/1 -> Function Call Generator VCU1/1
  VCU1Updated/1(signal_out) -> Logical Operator13/2
  [VINP_CANMsgValidVCU1_flg] -> Logical Operator13/1
  [VINP_CANMsgValidVCU1_flg] -> VCU1Updated/2(diagnosis_switch)
  Data Store Read35/1 -> Logical Operator14/1
  Data Store Read34/1 -> Logical Operator14/2
  Logical Operator14/1 -> VCU1Updated/3(Active_fault_flg)
  Function Call Generator VCU1/1 -> RollCntDiag_VCU1/Trigger
  Bus Selector1/7 -> RollCntDiag_VCU1/1  "<VHAL_VCU1RollCnt_cnt>"
  Logical Operator13/1 -> inputs_bus2/71  "VINP_CANMsgUpdatedVCU1_flg"
  VCU1Updated/2(signal_valid_out) -> inputs_bus2/72  "VINP_CANMsgVCU1RolCntOk_flg"
  RollCntDiag_VCU2/1 -> VCU2Updated/1(signal_in)
  Constant8/1 -> Function Call Generator VCU2/1
  VCU2Updated/1(signal_out) -> Logical Operator15/2
  [VINP_CANMsgValidVCU2_flg] -> Logical Operator15/1
  [VINP_CANMsgValidVCU2_flg] -> VCU2Updated/2(diagnosis_switch)
  Data Store Read37/1 -> Logical Operator16/1
  Data Store Read36/1 -> Logical Operator16/2
  Logical Operator16/1 -> VCU2Updated/3(Active_fault_flg)
  Function Call Generator VCU2/1 -> RollCntDiag_VCU2/Trigger
  Bus Selector1/8 -> RollCntDiag_VCU2/1  "<VHAL_VCU2RollCnt_cnt>"
  Logical Operator15/1 -> inputs_bus2/73  "VINP_CANMsgUpdatedVCU2_flg"
  VCU2Updated/2(signal_valid_out) -> inputs_bus2/74  "VINP_CANMsgVCU2RolCntOk_flg"
  [BHAL_outputs] -> Bus Selector/1
  Bus Selector/1 -> ABS2Checked/1(signal_in)  "<VHAL_ABS2ChkSumValid_flg>"
  Logical Operator17/1 -> inputs_bus2/77  "VINP_CANMsgCheckedABS2_flg"
  ABS2Checked/1(signal_out) -> Logical Operator17/2
  ABS2Checked/2(signal_valid_out) -> inputs_bus2/78  "VINP_CANMsgABS2ChkSumOk_flg"
  [VINP_CANMsgValidABS2_flg] -> Logical Operator17/1
  [VINP_CANMsgValidABS2_flg] -> ABS2Checked/2(diagnosis_switch)
  Data Store Read39/1 -> Logical Operator18/1
  Data Store Read38/1 -> Logical Operator18/2
  Logical Operator18/1 -> ABS2Checked/3(Active_fault_flg)
  Logical Operator20/1 -> inputs_bus2/79  "VINP_CANMsgCheckedSAS1_flg"
  Bus Selector/2 -> SAS1Checked/1(signal_in)  "<VHAL_SAS1ChkSumValid_flg>"
  SAS1Checked/1(signal_out) -> Logical Operator20/2
  SAS1Checked/2(signal_valid_out) -> inputs_bus2/80  "VINP_CANMsgSAS1ChkSumOk_flg"
  [VINP_CANMsgValidSAS1_flg] -> Logical Operator20/1
  [VINP_CANMsgValidSAS1_flg] -> SAS1Checked/2(diagnosis_switch)
  Data Store Read41/1 -> Logical Operator19/1
  Data Store Read40/1 -> Logical Operator19/2
  Logical Operator19/1 -> SAS1Checked/3(Active_fault_flg)
  Logical Operator22/1 -> inputs_bus2/81  "VINP_CANMsgCheckedEPAS2_flg"
  Bus Selector/3 -> EPAS2Checked/1(signal_in)  "<VHAL_EPAS2ChkSumValid_flg>"
  EPAS2Checked/1(signal_out) -> Logical Operator22/2
  EPAS2Checked/2(signal_valid_out) -> inputs_bus2/82  "VINP_CANMsgEPAS2ChkSumOk_flg"
  [VINP_CANMsgValidEPAS2_flg] -> Logical Operator22/1
  [VINP_CANMsgValidEPAS2_flg] -> EPAS2Checked/2(diagnosis_switch)
  Data Store Read43/1 -> Logical Operator21/1
  Data Store Read42/1 -> Logical Operator21/2
  Logical Operator21/1 -> EPAS2Checked/3(Active_fault_flg)
  Bus Selector/4 -> EPAS3Checked/1(signal_in)  "<VHAL_EPAS3ChkSumValid_flg>"
  EPAS3Checked/1(signal_out) -> Logical Operator23/2
  [VINP_CANMsgValidEPAS3_flg] -> Logical Operator23/1
  [VINP_CANMsgValidEPAS3_flg] -> EPAS3Checked/2(diagnosis_switch)
  Data Store Read45/1 -> Logical Operator24/1
  Data Store Read44/1 -> Logical Operator24/2
  Logical Operator24/1 -> EPAS3Checked/3(Active_fault_flg)
  Logical Operator23/1 -> inputs_bus2/83  "VINP_CANMsgCheckedEPAS3_flg"
  EPAS3Checked/2(signal_valid_out) -> inputs_bus2/84  "VINP_CANMsgEPAS3ChkSumOk_flg"
  Bus Selector/5 -> VCU1Checked/1(signal_in)  "<VHAL_VCU1ChkSumValid_flg>"
  VCU1Checked/1(signal_out) -> Logical Operator25/2
  [VINP_CANMsgValidVCU1_flg] -> Logical Operator25/1
  [VINP_CANMsgValidVCU1_flg] -> VCU1Checked/2(diagnosis_switch)
  Data Store Read47/1 -> Logical Operator26/1
  Data Store Read46/1 -> Logical Operator26/2
  Logical Operator26/1 -> VCU1Checked/3(Active_fault_flg)
  Logical Operator25/1 -> inputs_bus2/85  "VINP_CANMsgCheckedVCU1_flg"
  VCU1Checked/2(signal_valid_out) -> inputs_bus2/86  "VINP_CANMsgVCU1ChkSumOk_flg"
  Bus Selector/6 -> VCU2Checked/1(signal_in)  "<VHAL_VCU2ChkSumValid_flg>"
  VCU2Checked/1(signal_out) -> Logical Operator27/2
  [VINP_CANMsgValidVCU2_flg] -> Logical Operator27/1
  [VINP_CANMsgValidVCU2_flg] -> VCU2Checked/2(diagnosis_switch)
  Data Store Read49/1 -> Logical Operator28/1
  Data Store Read48/1 -> Logical Operator28/2
  Logical Operator28/1 -> VCU2Checked/3(Active_fault_flg)
  Logical Operator27/1 -> inputs_bus2/87  "VINP_CANMsgCheckedVCU2_flg"
  VCU2Checked/2(signal_valid_out) -> inputs_bus2/88  "VINP_CANMsgVCU2ChkSumOk_flg"
  HCU4Valid/1(signal_out) -> inputs_bus2/47  "VINP_CANMsgValidHCU4_flg"
  HCU4Valid/2(signal_valid_out) -> inputs_bus2/48  "VINP_CANMsgHCU4CommuOk_flg"
  Bus Selector5/24 -> HCU4Valid/1(signal_in)  "<VHAL_CANMsgValidHCU4_flg>"
  Data Store Read50/1 -> HCU4Valid/2(Active_fault_flg)
  HMI1Valid/1(signal_out) -> inputs_bus2/51  "VINP_CANMsgValidHMI1_flg"
  HMI1Valid/2(signal_valid_out) -> inputs_bus2/52  "VINP_CANMsgHMI1CommuOk_flg"
  Bus Selector5/26 -> HMI1Valid/1(signal_in)  "<VHAL_CANMsgValidHMI1_flg>"
  Data Store Read51/1 -> HMI1Valid/2(Active_fault_flg)
  HMI2Valid/1(signal_out) -> inputs_bus2/49  "VINP_CANMsgValidHMI2_flg"
  HMI2Valid/2(signal_valid_out) -> inputs_bus2/50  "VINP_CANMsgHMI2CommuOk_flg"
  Bus Selector5/25 -> HMI2Valid/1(signal_in)  "<VHAL_CANMsgValidHMI2_flg>"
  Data Store Read52/1 -> HMI2Valid/2(Active_fault_flg)
  Constant9/1 -> Function Call Generator HCU3/1
  Function Call Generator HCU3/1 -> RollCntDiag_HCU3/Trigger
  Bus Selector1/9 -> RollCntDiag_HCU3/1  "<VHAL_HCU3RollCnt_cnt>"
  RollCntDiag_HCU3/1 -> HCU3Updated/1(signal_in)
  HCU3Updated/1(signal_out) -> Logical Operator29/2
  [VINP_CANMsgValidHCU3_flg] -> Logical Operator29/1
  [VINP_CANMsgValidHCU3_flg] -> HCU3Updated/2(diagnosis_switch)
  Data Store Read59/1 -> Logical Operator30/1
  Data Store Read58/1 -> Logical Operator30/2
  Logical Operator30/1 -> HCU3Updated/3(Active_fault_flg)
  Logical Operator29/1 -> inputs_bus2/75  "VINP_CANMsgUpdatedHCU3_flg"
  HCU3Updated/2(signal_valid_out) -> inputs_bus2/76  "VINP_CANMsgHCU3RolCntOk_flg"
  Data Store Read60/1 -> EPAS1Valid/2(Active_fault_flg)
  EPAS1Valid/1(signal_out) -> inputs_bus2/23  "VINP_CANMsgValidEPAS1_flg"
  EPAS1Valid/2(signal_valid_out) -> inputs_bus2/24  "VINP_CANMsgEPAS1CommuOk_flg"
  Bus Selector5/12 -> EPAS1Valid/1(signal_in)  "<VHAL_CANMsgValidEPAS1_flg>"
  CSW1Valid/1(signal_out) -> inputs_bus2/53  "VINP_CANMsgValidCSW1_flg"
  CSW1Valid/2(signal_valid_out) -> inputs_bus2/54  "VINP_CANMsgCSW1CommuOk_flg"
  Data Store Read61/1 -> CSW1Valid/2(Active_fault_flg)
  Bus Selector5/27 -> CSW1Valid/1(signal_in)  "<VHAL_CANMsgValidCSW1_flg>"
  [BHAL_outputs] -> EPMValid/1(BHAL_outputs)
  EPMValid/1(VINP_CANMsgValidEPM_flg) -> inputs_bus2/55  "VINP_CANMsgValidEPM_flg"
  EPMValid/2(VINP_CANMsgEPMCommuOk_flg) -> inputs_bus2/56  "VINP_CANMsgEPMCommuOk_flg"
  [BHAL_outputs] -> EPMCheckReceived/1(BHAL_outputs)
  EPMCheckReceived/1(VINP_CamCANReceiveValid_flg) -> inputs_bus2/57  "VINP_CamCANReceiveValid_flg"
  EPMCheckReceived/2(VINP_CamCANReceiveOk_flg) -> inputs_bus2/58  "VINP_CamCANReceiveOk_flg"

== system inp/INP/CAN_Signals_Protected ==
  BHAL_outputs : Inport
  CANvalid_bus : Inport  Port=2
  Ground : Ground
  Terminator : Terminator
  Terminator1 : Terminator
  ProtCC_bus : Outport
  wires:
  Ground/1 -> ProtCC_bus/1  "ProtCC_bus"
  CANvalid_bus/1 -> [CANvalid_bus]
  BHAL_outputs/1 -> [BHAL_outputs]
  [BHAL_outputs] -> Terminator1/1
  [CANvalid_bus] -> Terminator/1

== system inp/INP/CSW_signals ==
  BHAL_outputs : Inport  OutDataTypeStr=Bus: BHAL_outputs
  CANvalid_bus : Inport  Port=2
  Bus Selector1 : BusSelector  OutputSignals=VHAL_RearFogSwOn_flg
  Bus Selector2 : BusSelector  OutputSignals=VINP_CANMsgValidCSW1_flg
  Bus Selector3 : BusSelector  OutputSignals=VHAL_HighBeamSwOn_flg
  Bus Selector4 : BusSelector  OutputSignals=VINP_CANMsgValidCSW1_flg
  Constant : Constant  Value=TRUE
  Constant1 : Constant  Value=TRUE
  Data Store Read1 : DataStoreRead  DataStoreName=VDDML_CSW1CANCommuFault_AF_flg
  Data Store Read2 : DataStoreRead  DataStoreName=VDDML_CSW1CANCommuFault_AF_flg
  Data Type Conversion : DataTypeConversion  OutDataTypeStr=boolean
  Data Type Conversion1 : DataTypeConversion  OutDataTypeStr=boolean
  Terminator{,1..3} (4 alike) : Terminator
  inputs_bus2 : BusCreator
  CSW_signal_bus : Outport
  wires:
  inputs_bus2/1 -> CSW_signal_bus/1
  BHAL_outputs/1 -> [BHAL_outputs]
  CANvalid_bus/1 -> [CANvalid_bus]
  Bus Selector1/1 -> Data Type Conversion/1  "<VHAL_RearFogSwOn_flg>"
  [BHAL_outputs] -> Bus Selector1/1
  Data Type Conversion/1 -> inputs_bus2/1  "VINP_RearFogSwOn_flg_InHAL"
  Constant/1 -> inputs_bus2/2  "VINP_RearFogSwOnValid_flg_InHAL"
  Bus Selector2/1 -> Terminator/1  "<VINP_CANMsgValidCSW1_flg>"
  [CANvalid_bus] -> Bus Selector2/1
  Data Store Read1/1 -> Terminator1/1
  Bus Selector3/1 -> Data Type Conversion1/1  "<VHAL_HighBeamSwOn_flg>"
  [BHAL_outputs] -> Bus Selector3/1
  Data Type Conversion1/1 -> inputs_bus2/3  "VINP_HighBeamSwOn_flg_InHAL"
  Constant1/1 -> inputs_bus2/4  "VINP_HighBeamSwOnValid_flg_InHAL"
  Bus Selector4/1 -> Terminator2/1  "<VINP_CANMsgValidCSW1_flg>"
  [CANvalid_bus] -> Bus Selector4/1
  Data Store Read2/1 -> Terminator3/1
